simulink model "MCVT_Integration"  (R2020a)
settings: Solver=ode8  StartTime=0.0  StopTime=T_end  FixedStep=dt  RelTol=1e-3  EnableMultiTasking=on
values from neighbouring files (symbols this model uses):
  assumed_power_voltage = 1.25e2    (= 125)    [MCVT_Input_File.m]    (also in MCVT_Run_QuickCheck.m)
  battery_power_toggle = 1    [MCVT_Input_File.m]    (also in MCVT_Run_QuickCheck.m)
  battery_trickle_charge_param = 0.1    [MCVT_Input_File.m]    (also in MCVT_Run_QuickCheck.m)
  battery_trickle_leakage_param = 0.01    [MCVT_Input_File.m]    (also in MCVT_Run_QuickCheck.m)
  damage_case_id = 3    [MCVT_Input_File.m]    (also in MCVT_Run_QuickCheck.m = 1)
  dt = 1/fs    (= 0.001)    [MCVT_Run_QuickCheck.m]    (also in MCVT_Sim_Set.m)
  dt_2 = 1/fs_2    (= 0.001)    [MCVT_Run_QuickCheck.m]    (also in MCVT_Sim_Set.m)
  dt_3 = 1/fs_3    (= 0.001)    [MCVT_Run_QuickCheck.m]    (also in MCVT_Sim_Set.m)
  dt_5 = 1/fs_5    (= 0.005)    [MCVT_Run_QuickCheck.m]    (also in MCVT_Sim_Set.m)
  dt_8 = 1/fs_8    (= 0.05)    [MCVT_Run_QuickCheck.m]    (also in MCVT_Sim_Set.m)
  dt_9 = 1/fs_9    (= 0.001)    [MCVT_Run_QuickCheck.m]    (also in MCVT_Sim_Set.m)
  dust_met_duration = 3    [MCVT_Input_File.m]    (also in MCVT_Run_QuickCheck.m = 2)
  ECLSS_indoor_set_temperature = 298.15    [MCVT_Input_File.m]    (also in MCVT_Run_QuickCheck.m)
  energy_store_max_o = 150    [MCVT_Input_File.m]    (also in MCVT_Run_QuickCheck.m)
  energy_store_o = 120    [MCVT_Input_File.m]    (also in MCVT_Run_QuickCheck.m)
  fs = 1000    [MCVT_Run_QuickCheck.m]    (also in MCVT_Sim_Set.m)
  gain_charge_param = 1    [MCVT_Input_File.m]    (also in MCVT_Run_QuickCheck.m)
  ground_temperature = 373    [MCVT_Habitat_Design_Input_File.m]    (also in MCVT_Run_QuickCheck.m)
  I_max_charge = 800    [MCVT_Input_File.m]    (also in MCVT_Run_QuickCheck.m)
  I_max_discharge = 800    [MCVT_Input_File.m]    (also in MCVT_Run_QuickCheck.m)
  initial_pressure = 1e5    (= 100000)    [MCVT_Input_File.m]    (also in MCVT_Run_QuickCheck.m)
  initial_temperature = 295    [MCVT_Input_File.m]    (also in MCVT_Run_QuickCheck.m)
  interior_leak_rate = 0.00415    [MCVT_Input_File.m]    (also in MCVT_Run_QuickCheck.m)
  launch_dust_accum_start_time = 10    [MCVT_Input_File.m]    (also in MCVT_Run_QuickCheck.m = 2)
  launch_dust_accum_stop_time = 12    [MCVT_Input_File.m]    (also in MCVT_Run_QuickCheck.m = 3)
  meteorite_impact_time = 2    [MCVT_Input_File.m]    (also in MCVT_Run_QuickCheck.m = 1)
  nuclear_power_toggle = 1    [MCVT_Input_File.m]    (also in MCVT_Run_QuickCheck.m)
  print_interval = 30    [MCVT_Input_File.m]
  SOC_lower_thres = 0.5    [MCVT_Input_File.m]    (also in MCVT_Run_QuickCheck.m)
  SOC_upper_thres = 0.9    [MCVT_Input_File.m]    (also in MCVT_Run_QuickCheck.m)
  solar_power_toggle = 1    [MCVT_Input_File.m]    (also in MCVT_Run_QuickCheck.m)
  space_temperature = 4    [MCVT_Habitat_Design_Input_File.m]    (also in MCVT_Run_QuickCheck.m)
  Start_angle = 90    [MCVT_Input_File.m]    (also in MCVT_Run_QuickCheck.m)
undefined symbols (not defined in the file or in its folder): A_flow_restrictor, ACTIVITY_LOOKUP_TABLE, air, Aref, Bmap, Bmap2, Bmap3, comms, DiscIntNeverNeededParam, DmgSPL, dust_parameters, ECLSS_dust_damage_repair_time, ECLSS_heater_capacity, ECLSS_IPS_restrictor, ECLSS_IPS_setpointPressure, ECLSS_paint_damage_repair_time, ECLSS_radiatorLoop_discreteNumber, ECLSS_Vst, emptyActivity, expected_heat_add, FI, habitat, HIEM2SMM, HIEM2STM, HIEMDamageElements, impact_numbering, initial_SPLT_damage_index, input_air, input_Cond, input_CP, input_discretization, input_EV, input_Evap, input_radiatorLoop, K_ECLSS_dust_single, K_ECLSS_paint_single, K_Structure_damage_single, Kref, kW_to_W, meteoroidDustDep, mFRF, ndelays, nominalDustDep, noverlap, nuclear_toggle_gain, output_limits, panel_paint0, Ps0, reference_temperature, RepairTime_ECLSS_factor, Sensor_Location_SMM, SMM_T0, SMM_T1, SMM_T2, SMM_T3, SMM_T4, SMM_T4_0, SMM_T4_1, SMM_T4_2, solar_FDD_K ... (+73)
libraries: neural x350, simulink x39, xpcutilitieslib x7, xpcrtudplib x7, dspstat3 x1
(part 2 of 43)

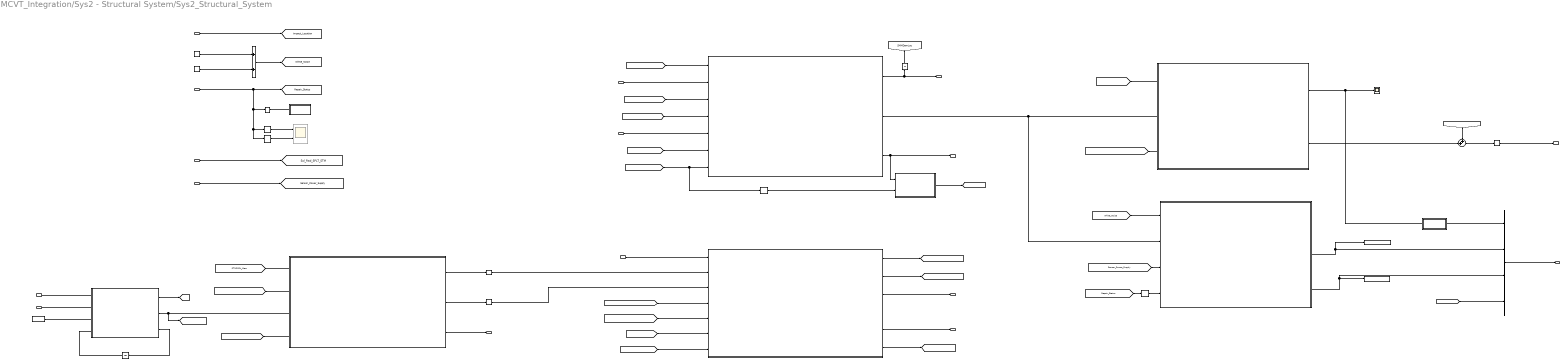
== system MCVT_Integration/Sys2 - Structural System/Sys2_Structural_System ==
  note: Structural Protective Layer (2.4) - Soon it will become its own subsystem block
  note: FDD: Fault Detection and Diagnosis (2.3)
  note: Structural Thermal Model (2.2)
  note: Structural Mechanical Model (2.1)
  note: Input Distribution
  Sys2_In_Solar_Irradiation [W/m^2] : Inport
  Sys2_In_Moonquake_Acceleration [m/s^2] : Inport  Port=2
  Sys2_In_Meteoroid_Location [m] : Inport  Port=3
  Sys2_In_FI_Index [-] : Inport  Port=4
  Sys2_In_dmg_case [-] : Inport  Port=5
  Sys2_In_Interior_Surface_Temperature [K] : Inport  Port=6
  Sys2_In_Internal_Pressure [Pa] : Inport  Port=7
  Sys2_In_Power_Supply [kW] : Inport  Port=8
  Sys2_In_Repair_Status [-] : Inport  Port=9
  3D Structural Protective Layer-Thermal (SPL-T) : Subsystem
  Acc Excitation : simulink/Sources/Band-Limited White Noise  Cov=[1e-9]  Ts=Time_step  seed=[23341]
  Add : Sum
  Bus Creator : BusCreator  Inputs=4
  Derivative1 : Derivative
  FDD adjustment : Subsystem
  Fault Detection and Diagnosis (FDD) 2.3 : Subsystem
  Fault Detection and Diagnosis (FDD) with Binary Classifer : Subsystem
  Impact_Force_&_SPL_Damage : MATLAB Function  TreatAsAtomicUnit=on
  MemBlock : Memory  InitialCondition=initial_SPLT_damage_index
  Memory : Memory  InitialCondition=0.0036
  Repair Status : Constant  Value=0
  SPL2STM_Mem : Memory  InitialCondition=293.15.*ones(10,6)
  STM2SPL_Mem : Memory  InitialCondition=293.15.*ones(10,6)
  Scope4 : Scope
  Selector : Selector  InputPortWidth=4  IndexOptions=Index vector (dialog)  Indices=1  OutputSizes=1
  Selector1 : Selector  InputPortWidth=4  IndexOptions=Index vector (dialog)  Indices=2  OutputSizes=1
  Selector2 : Selector  InputPortWidth=4  IndexOptions=Index vector (dialog)  Indices=3  OutputSizes=1
  Selector3 : Selector  InputPortWidth=4  IndexOptions=Index vector (dialog)  Indices=idx_Activity_Completion_Flag  OutputSizes=1
  Sim FDD Damage Detection : Subsystem
  Structural Mechanical 2.1 : Subsystem
  Structural Thermal Model 2.2 : Subsystem
  Sum : Sum  Inputs=++|
  To Workspace : ToWorkspace  VariableName=DamageProbabilities  SaveFormat=Timeseries  SampleTime=-1
  To Workspace1 : ToWorkspace  VariableName=ImpactProbabilities  SaveFormat=Timeseries  SampleTime=-1
  Unit Delay : UnitDelay  InitialCondition=zeros(324,1)  SampleTime=-1  HasFrameUpgradeWarning=on
  Unit Delay1 : UnitDelay  SampleTime=-1  HasFrameUpgradeWarning=on
  White Noise : simulink/Sources/Band-Limited White Noise  Cov=Noise_power  Ts=Time_step  seed=[23341]
  printRepair : MATLAB Function  TreatAsAtomicUnit=on
  repair : Scope  Commented=on  NumInputPorts=2
  Sys2_out_InteriorWallSurfaceTemp_Sensors [K] : Outport
  Sys2_Out_Damage_Information [-] : Outport  Port=2
  Sys2_out_PowerConsumption [kW] : Outport  Port=3
  Sys2_out_InteriorWallSurfaceTemp [K] : Outport  Port=4  PortDimensions=[60 1]
  Sys2_out_Damage [-] : Outport  Port=5
  Sys2_out_ExteriorWallTemp [K] : Outport  Port=6
  Sys2_out_Total_Damage [-] : Outport  Port=7
  tag scope: ImpForce=global, Impact_Location=global, Repair_Status=global, SMMDamLoc=global, SPL_Dam=global, STM2SMM=global, STM2SPL_Mem=global, Sensor_Power_Supply=global, Sol_Rad_SPLT_STM=global, Tot_Pow_Con=global, White_Noise=global
  wires:
  Acc Excitation/1 -> Add/1
  White Noise/1 -> Add/2
  Structural Mechanical 2.1/2(Sys2.1_Out_Acceleration) -> Fault Detection and Diagnosis (FDD) with Binary Classifer/2(Sys2.3_In_Acceleration Response), Fault Detection and Diagnosis (FDD) 2.3/2(Sys2.3_In_Acceleration Response)
  Sys2_In_Internal_Pressure [Pa]/1 -> Structural Mechanical 2.1/2(Sys2.1_In_Internal_Pressure)
  Sys2_In_Moonquake_Acceleration [m/s^2]/1 -> Structural Mechanical 2.1/5(Sys2.1_In_Moonquake_Acceleration)
  Sum/1 -> Memory/1
  Fault Detection and Diagnosis (FDD) 2.3/1(Sys2.3_Out_Probabilities) -> FDD adjustment/1(x), Scope4/1
  Fault Detection and Diagnosis (FDD) 2.3/2(Sys2.3_Out_Accelerometer Power Consumption (kW)) -> Sum/2
  Structural Mechanical 2.1/1(Sys2.1_Out_Damage_Locations) -> Sys2_out_Damage [-]/1, Unit Delay/1
  Sys2_In_FI_Index [-]/1 -> Impact_Force_&_SPL_Damage/1(FI_index)
  Sys2_In_dmg_case [-]/1 -> Impact_Force_&_SPL_Damage/2(dmg_case)
  Impact_Force_&_SPL_Damage/3(DmgHist) -> Unit Delay1/1
  Unit Delay1/1 -> Impact_Force_&_SPL_Damage/4("DmgHist ")
  Repair Status/1 -> Impact_Force_&_SPL_Damage/3(repair_status)
  Derivative1/1 -> printRepair/1(x)
  Memory/1 -> Sys2_out_PowerConsumption [kW]/1
  Structural Mechanical 2.1/3(Sys2.1_Out_Total_Damage) -> Sim FDD Damage Detection/1(Sys2_Total_Damage), Sys2_out_Total_Damage [-]/1
  FDD adjustment/1(y) -> Bus Creator/1  "Sys2_Out_PanelDamageProbabilities"
  Bus Creator/1 -> Sys2_Out_Damage_Information [-]/1  "Sys2_Out_Damage_Information_to_Sys6"
  Fault Detection and Diagnosis (FDD) with Binary Classifer/3(Sys2.3_Out_Impact_location_Probabilities) -> To Workspace1/1, Bus Creator/3  "Sys2_Out_Impact_Location_Probabiilities"
  Fault Detection and Diagnosis (FDD) with Binary Classifer/2(Sys2.3_Out_Damage_Detection_Probabilities) -> To Workspace/1, Bus Creator/2  "Sys2_Out_Damage_Detection_Probabilities"
  Selector/1 -> Fault Detection and Diagnosis (FDD) with Binary Classifer/4(Sys2.3_In_Repair_Status)
  Sys2_In_Repair_Status [-]/1 -> Selector2/1, Selector1/1, Derivative1/1, [Repair_Status]
  Selector1/1 -> repair/1
  Selector2/1 -> repair/2
  Sys2_In_Meteoroid_Location [m]/1 -> [Impact_Location]
  Structural Thermal Model 2.2/3(Sys2.2_out_InteriorWallSurfTemp_Sensors) -> Sys2_out_InteriorWallSurfaceTemp_Sensors [K]/1
  Structural Thermal Model 2.2/5(Sys2.2_out_InteriorWallSurfaceTemp_(to_Sys8)) -> Sys2_out_InteriorWallSurfaceTemp [K]/1
  3D Structural Protective Layer-Thermal (SPL-T)/1(Sys2.4_SPL2STM_MemoryBlock) -> SPL2STM_Mem/1
  3D Structural Protective Layer-Thermal (SPL-T)/3(Sys2.4_out_ExteriorWallTemp) -> Sys2_out_ExteriorWallTemp [K]/1
  Sys2_In_Interior_Surface_Temperature [K]/1 -> Structural Thermal Model 2.2/1(Sys2.2_In_Interior_Surface_Temperature_(from_Sys8))
  Unit Delay/1 -> [SMMDamLoc]
  [SMMDamLoc] -> Structural Thermal Model 2.2/7(Sys2.2_In_HabitatWallDamage)
  Structural Thermal Model 2.2/6(Sys2.2_out_WallTempDistribution_toSMM (To Sys 2.1)) -> [STM2SMM]
  [STM2SMM] -> Structural Mechanical 2.1/1(Sys2.1_In_Temp_Profile)
  Impact_Force_&_SPL_Damage/2(Dmg) -> 3D Structural Protective Layer-Thermal (SPL-T)/3(Sys2.4_In_Damage_SPL), [SPL_Dam]
  [SPL_Dam] -> Structural Thermal Model 2.2/6(Sys2.2_In_Damage_SPL)
  [Impact_Location] -> 3D Structural Protective Layer-Thermal (SPL-T)/4(Sys2.4_In_Meteorite_Impact_Location)
  Impact_Force_&_SPL_Damage/1(Fimp) -> [ImpForce]
  [ImpForce] -> Structural Mechanical 2.1/3(Sys2.1_In_Impact_Force)
  Sys2_In_Power_Supply [kW]/1 -> [Sensor_Power_Supply]
  [Sensor_Power_Supply] -> Structural Thermal Model 2.2/5(Sys2.2_In_SensorPowerSupply)
  [Sensor_Power_Supply] -> Fault Detection and Diagnosis (FDD) 2.3/3(Sys2.3_In_Accelerometer Power Supply (kW))
  [Sensor_Power_Supply] -> Fault Detection and Diagnosis (FDD) with Binary Classifer/3(Sys2.3_In_Accelerometer Power Supply (kW))
  3D Structural Protective Layer-Thermal (SPL-T)/2(Sys2.4_DamageElementNumber_SPLT_to_STM) -> MemBlock/1
  Sys2_In_Solar_Irradiation [W/m^2]/1 -> [Sol_Rad_SPLT_STM]
  [Sol_Rad_SPLT_STM] -> Structural Thermal Model 2.2/4(Sys2.2_In_Solar_Irradiation_(from_Sys9))
  [Sol_Rad_SPLT_STM] -> 3D Structural Protective Layer-Thermal (SPL-T)/2(Sys2.4_In_Solar_Irradiation_(from_Sys9))
  Structural Thermal Model 2.2/2(Sys2.2_STM2SPL_Mem) -> [STM2SPL_Mem]
  [STM2SPL_Mem] -> 3D Structural Protective Layer-Thermal (SPL-T)/1(Sys2.4_STM2SPL_MemoryBock)
  SPL2STM_Mem/1 -> Structural Thermal Model 2.2/2(Sys2.2_SPL2STM_Mem)
  [Tot_Pow_Con] -> Sum/1
  Structural Thermal Model 2.2/1(Sys2.2_out_TotalPowerConsumption (kW)) -> [Tot_Pow_Con]
  Add/1 -> [White_Noise]
  [White_Noise] -> Structural Mechanical 2.1/6(Sys2.1_In_White Noise)
  [White_Noise] -> Fault Detection and Diagnosis (FDD) 2.3/1(Sys2.3_In_Excitation (White Noise))
  [White_Noise] -> Fault Detection and Diagnosis (FDD) with Binary Classifer/1(Sys2.3_In_Excitation (White Noise))
  [Repair_Status] -> Selector3/1, Structural Mechanical 2.1/7(Sys2.1_In_Repair_Status)
  [Repair_Status] -> Selector/1
  [Impact_Location] -> Structural Mechanical 2.1/4(Sys_2.1_In_Impact_Location)
  MemBlock/1 -> Structural Thermal Model 2.2/3(Sys2.2_Damage_Index_SPLT)
  Selector3/1 -> Sim FDD Damage Detection/2(EnableResetFDD_Structure)
  Sim FDD Damage Detection/1(FDD_Structure_Damage_xe) -> [FDD_Structure_Damage_xe]  "FDD_Structure_Damage_xe"
  [FDD_Structure_Damage_xe] -> Bus Creator/4  "Sys2_Out_FDD_Structure_Damage_xe"

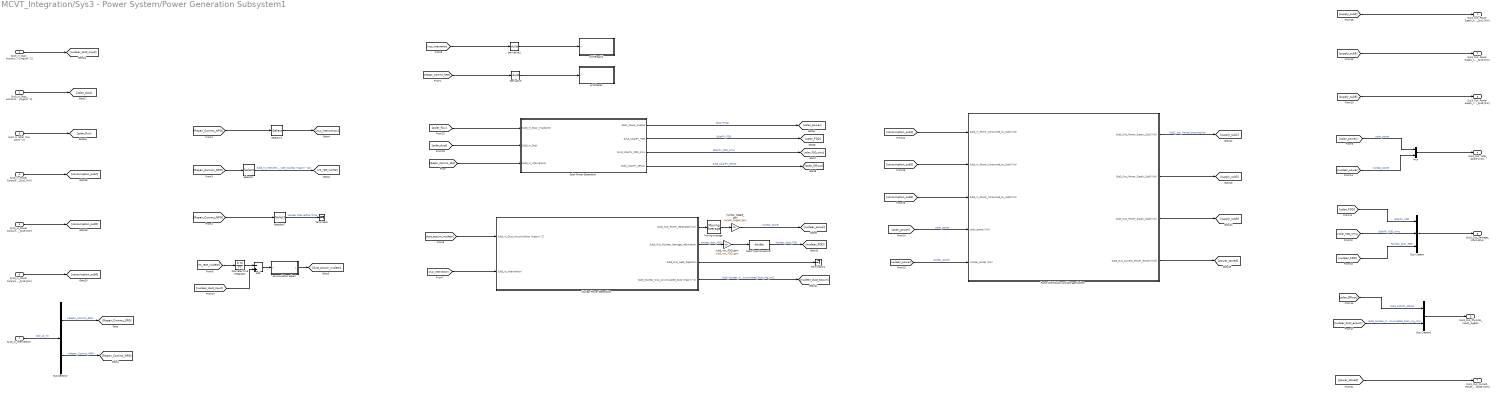
== system MCVT_Integration/Sys3 - Power System/Power Generation Subsystem1 ==
  Sys3_In_Dust_Accumulation_Nuclear [mg/cm^2] : Inport
  Sys3_In_Dust_Accumulation_PV [mg/cm^2] : Inport  Port=2
  Sys3_In_Solar_Flux [W/m^2] : Inport  Port=3
  Sys3_In_Power_Consumed_by_Sys2 [kW] : Inport  Port=4
  Sys3_In_Power_Consumed_by_Sys5 [kW] : Inport  Port=5
  Sys3_In_Power_Consumed_by_Sys8 [kW] : Inport  Port=6
  Sys3_In_Intervention : Inport  Port=7
  Add : Sum  Inputs=-+
  Bus Creator : BusCreator  Inputs=3
  Bus Creator1 : BusCreator  Inputs=2
  Bus Selector : BusSelector  OutputSignals=Repair_Comms_SPG,Repair_Comms_NPG
  Data Type Conversion : DataTypeConversion  OutDataTypeStr=double
  Derivative : Derivative
  Derivative1 : Derivative
  Discrete-Time Integrator : DiscreteIntegrator  SampleTime=-1  ICPrevOutput=DiscIntNeverNeededParam  ICPrevScaledInput=DiscIntNeverNeededParam
  Moving Average : dspstat3/Moving Average  Method=Sliding window  ForgettingFactorPort=off  SpecifyWindowLength=on  WindowLength=1000  ForgettingFactor=0.9  SimulateUsing=Code generation
  Mux : Mux  Inputs=2
  Nuclear Power Generation : Subsystem
  Power Distribution and Storage System : Subsystem
  Selector : Selector  InputPortWidth=4  IndexOptions=Index vector (dialog)  Indices=idx_repair_action_rate  OutputSizes=1
  Selector1 : Selector  InputPortWidth=4  IndexOptions=Index vector (dialog)  Indices=idx_failure_mode  OutputSizes=1
  Selector2 : Selector  InputPortWidth=4  IndexOptions=Index vector (dialog)  Indices=idx_repair_action_time  OutputSizes=1
  Solar Power Generation : Subsystem
  Terminator : Terminator
  Terminator1 : Terminator
  accumulation adjust : MATLAB Function  TreatAsAtomicUnit=on  SystemSampleTime=dt_3
  nuclear_toggle_gain : Gain  Gain=nuclear_toggle_gain
  printRepair : MATLAB Function  TreatAsAtomicUnit=on
  printRepair1 : MATLAB Function  TreatAsAtomicUnit=on
  sub3_nuc_FDD_gain : Gain  Gain=sub3_nuc_FDD_gain
  Sys3_Out_Power_Supply_to_Sys2 [kW] : Outport
  Sys3_Out_Power_Supply_to_Sys5 [kW] : Outport  Port=2
  Sys3_Out_Power_Supply_to_Sys8 [kW] : Outport  Port=3
  Sys3_Out_total_power [kW] : Outport  Port=4
  Sys3_Out_Physical_Inputs_Agents : Outport  Port=5
  Sys3_Out_Damage_Information : Outport  Port=6
  Sys3_Out_Current_Power_Stored [kWh] : Outport  Port=7
  wires:
  Sys3_In_Solar_Flux [W/m^2]/1 -> [solar_flux]
  Sys3_In_Power_Consumed_by_Sys5 [kW]/1 -> [consumption_sub5]
  [supply_sub5] -> Sys3_Out_Power_Supply_to_Sys5 [kW]/1
  Solar Power Generation/1(Solar_Power_Output) -> [solar_power]  "Solar Pmpp"
  Nuclear Power Generation/1(Sub3_Out_Power_Generated (kW)) -> Moving Average/1
  Nuclear Power Generation/3(Sub3_Out_Heat_Rejection) -> Terminator1/1
  Moving Average/1 -> nuclear_toggle_gain/1
  [nuclear_power] -> Mux/2  "nuclear_power"
  Bus Creator/1 -> Sys3_Out_Damage_Information/1
  [solar_FDD] -> Bus Creator/1  "SolarPV_FDD"
  Mux/1 -> Sys3_Out_total_power [kW]/1
  Sys3_In_Dust_Accumulation_PV [mg/cm^2]/1 -> [solar_dust]
  [solar_power] -> Mux/1  "solar_power"
  Bus Selector/1 -> [Repair_Comms_SPG]  "<Repair_Comms_SPG>"
  Derivative/1 -> printRepair/1(x)
  Derivative1/1 -> printRepair1/1(x)
  Sys3_In_Power_Consumed_by_Sys8 [kW]/1 -> [consumption_sub8]
  [supply_sub8] -> Sys3_Out_Power_Supply_to_Sys8 [kW]/1
  Sys3_In_Power_Consumed_by_Sys2 [kW]/1 -> [consumption_sub2]
  [supply_sub2] -> Sys3_Out_Power_Supply_to_Sys2 [kW]/1
  [power_stored] -> Sys3_Out_Current_Power_Stored [kWh]/1
  Nuclear Power Generation/2(Sub3_Out_Nuclear_Damage_Information) -> sub3_nuc_FDD_gain/1  "nuclear_dust_FDD"
  [nuclear_FDD] -> Bus Creator/3  "Nuclear_Dust_FDD"
  sub3_nuc_FDD_gain/1 -> Data Type Conversion/1
  Sys3_In_Dust_Accumulation_Nuclear [mg/cm^2]/1 -> [nuclear_dust_input]
  [nuc_intervenion] -> Nuclear Power Generation/2(Sub3_In_Intervention)
  accumulation adjust/1(y) -> [dust_accum_nuclear]
  [solar_DRout] -> Bus Creator1/1  "Sub3_solarPV_DRout"
  Bus Creator1/1 -> Sys3_Out_Physical_Inputs_Agents/1
  [nuclear_dust_accum] -> Bus Creator1/2  "Sub3_Nuclear_Out_Accumulated_Dust_mg_cm2"
  Selector/1 -> [Int_rate_nuclear]  "Sub3_In_Intervention_rate_Nuclear (mg/cm^2/s)"
  [Repair_Comms_NPG] -> Selector2/1
  Sys3_In_Intervention/1 -> Bus Selector/1  "look_at_int"
  [solar_FDD_simu] -> Bus Creator/2  "SolarPV_FDD_simu"
  Selector2/1 -> Terminator/1  "Nuclear Intervention Time"
  Discrete-Time Integrator/1 -> Add/1
  Add/1 -> accumulation adjust/1(x)
  [Repair_Comms_SPG] -> Solar Power Generation/3(Sub3_In_intervension)
  [Repair_Comms_SPG] -> Derivative/1
  Bus Selector/2 -> [Repair_Comms_NPG]  "<Repair_Comms_NPG>"
  [Repair_Comms_NPG] -> Selector/1
  [Repair_Comms_NPG] -> Selector1/1
  [Int_rate_nuclear] -> Discrete-Time Integrator/1
  [dust_accum_nuclear] -> Nuclear Power Generation/1(Sub3_In_Dust_Accumulation (mg/cm^2))
  Selector1/1 -> [nuc_intervenion]
  [nuc_intervenion] -> Derivative1/1
  [solar_power] -> Power Distribution and Storage System/4(solar_power (kW))  "solar_power"
  nuclear_toggle_gain/1 -> [nuclear_power]  "nuclear_power"
  [nuclear_power] -> Power Distribution and Storage System/5(nuclear_power (kW))  "nuclear_power"
  Data Type Conversion/1 -> [nuclear_FDD]  "Nuclear_Dust_FDD"
  Nuclear Power Generation/4(Sub3_Nuclear_Out_Accumulated_Dust (mg/cm^2)) -> [nuclear_dust_accum]  "Sub3_Nuclear_Out_Accumulated_Dust_mg_cm2"
  Power Distribution and Storage System/1(Sub3_Out_Power_Supply_Sub2 (kW)) -> [supply_sub2]  "Sub2_ out_ PowerConsumpstion"
  Power Distribution and Storage System/2(Sub3_Out_Power_Supply_Sub5 (kW)) -> [supply_sub5]
  Power Distribution and Storage System/3(Sub3_Out_Power_Supply_Sub8 (kW)) -> [supply_sub8]
  Power Distribution and Storage System/4(Sub3_Out_Current_Power_Stored (kWh)) -> [power_stored]
  [solar_flux] -> Solar Power Generation/1(Sub3_In_Solar_Irradiance)
  [solar_dust] -> Solar Power Generation/2(Sub3_In_Dust)
  [consumption_sub2] -> Power Distribution and Storage System/1(Sub3_In_Power_Consumed_by_Sub2 (kW))
  [consumption_sub5] -> Power Distribution and Storage System/2(Sub3_In_Power_Consumed_by_Sub5 (kW))
  [consumption_sub8] -> Power Distribution and Storage System/3(Sub3_In_Power_Consumed_by_Sub8 (kW))
  [nuclear_dust_input] -> Add/2
  Solar Power Generation/2(Sys3_solarPV_FDD) -> [solar_FDD]  "SolarPV_FDD"
  Solar Power Generation/3(Sys3_solarPV_FDD_simu) -> [solar_FDD_simu]  "SolarPV_FDD_simu"
  Solar Power Generation/4(Sub3_solarPV_DRout) -> [solar_DRout]  "Sub3_solarPV_DRout"

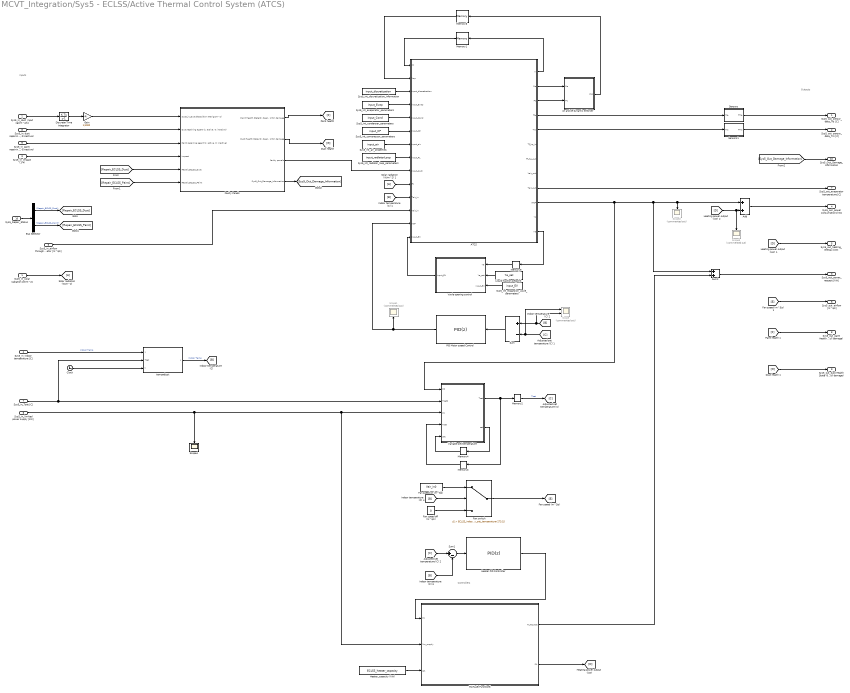
== system MCVT_Integration/Sys5 - ECLSS/Active Thermal Control System (ATCS) ==
  note: Inputs
  note: Outputs
  note: Controllers
  Sys5_in_solar radiation [W/m^2] : Inport
  Sys5_in_dust_Input [g/cm^2/s] : Inport  Port=2
  Sys5_in_impact (1-4) : Inport  Port=3
  Sys5_in_indoor temperature [C] : Inport  Port=4
  Sys5_in_airflow through the evaporator [m^3/s] : Inport  Port=5
  Sys5_in_limited power supply [kW] : Inport  Port=6
  Sys5_in_Tset [C] : Inport  Port=7
  "Sys5_in_dust repairing agent (1:active, 0:inactive)" : Inport  Port=8
  "Sys5_in_paint repairing agent (1:active, 0:inactive)" : Inport  Port=9
  Sys5_Repair_Status : Inport  Port=10
  ATCS : MATLAB Function  TreatAsAtomicUnit=on  SystemSampleTime=dt_5
  Add : Sum
  Bus Selector : BusSelector  OutputSignals=Repair_ECLSS_Dust,Repair_ECLSS_Paint
  Clock : Clock
  Discrete-Time Integrator : DiscreteIntegrator  SampleTime=-1  ICPrevOutput=DiscIntNeverNeededParam  ICPrevScaledInput=DiscIntNeverNeededParam
  Fan speed off (m^3/s) : Constant  Value=0  OutDataTypeStr=Inherit: Inherit via back propagation
  Fan speed on (m^3/s) : Constant  Value=Vair_in0
  Fan switch : Switch  Criteria=u2 > Threshold  Threshold=ECLSS_indoor_set_temperature-273.15
  Faulty Panels : Subsystem
  Gain : Gain  Gain=1/1000
  Heater PID Controller : simulink/Continuous/PID Controller  Controller=PID  Form=Parallel  TimeDomain=Discrete-time  UseExternalTs=off  SampleTime=dt_5  IntegratorMethod=Forward Euler  FilterMethod=Forward Euler  ControllerParametersSource=internal  P=30  I=5  D=0  UseFilter=on  N=100  TunerSelectOption=Transfer Function Based (PID Tuner App)  InitialConditionSource=internal  InitialConditionForIntegrator=0  InitialConditionForFilter=0  DifferentiatorICPrevScaledInput=0  ExternalReset=none  TrackingMode=off  Kt=1  LimitOutput=on  UpperSaturationLimit=inf  LowerSaturationLimit=0  LinearizeAsGain=on  AntiWindupMode=none  Kb=1  POutMin=[]  POutMax=[]  IOutMin=[]  IOutMax=[]  DOutMin=[]  DOutMax=[]  NOutMin=[]  NOutMax=[]  SumOutMin=[]  SumOutMax=[]  SaturationOutMin=[]  SaturationOutMax=[]  PParamMin=[]  PParamMax=[]  IParamMin=[]  IParamMax=[]  DParamMin=[]  DParamMax=[]  NParamMin=[]  NParamMax=[]  KbParamMin=[]  KbParamMax=[]  KtParamMin=[]  KtParamMax=[]  KbOutMin=[]  KbOutMax=[]  KtOutMin=[]  KtOutMax=[]  IntegratorOutMin=[]  IntegratorOutMax=[]  FilterOutMin=[]  FilterOutMax=[]  SumI1OutMin=[]  SumI1OutMax=[]  SumI2OutMin=[]  SumI2OutMax=[]  SumI3OutMin=[]  SumI3OutMax=[]  SumI4OutMin=[]  SumI4OutMax=[]  SumDOutMin=[]  SumDOutMax=[]  DifferentiatorOutMin=[]  DifferentiatorOutMax=[]  FilterDiffOutCoefMin=[]  FilterDiffOutCoefMax=[]  ReciprocalOutMin=[]  ReciprocalOutMax=[]  SumDenOutMin=[]  SumDenOutMax=[]  SumNumOutMin=[]  SumNumOutMax=[]  DivideOutMin=[]  DivideOutMax=[]  UdiffTsProdOutMin=[]  UdiffTsProdOutMax=[]  NTsProdOutMin=[]  NTsProdOutMax=[]  UintegralTsProdOutMin=[]  UintegralTsProdOutMax=[]  UngainTsProdOutMin=[]  UngainTsProdOutMax=[]  IntegratorContinuousStateAttributes=''  IntegratorStateMustResolveToSignalObject=off  IntegratorRTWStateStorageClass=Auto  FilterContinuousStateAttributes=''  FilterStateMustResolveToSignalObject=off  FilterRTWStateStorageClass=Auto  ParallelPVariant=InternalParameters  IdealPVariant=Disabled  IVariant=InternalParameters  DVariant=InternalParameters  IntegratorVariant=Discrete  SatVariant=Enabled  AWVariant=Passthrough  PCopyVariant=Disabled  TRVariant=Disabled  FdbkBlocksVariant=Forward  IdealPFdbkVariant=Disabled  SatFdbkVariant=Disabled  DerivativeFilterVariant=ForwardEulerFilter  NVariant=InternalParameters  NCopyVariant=Disabled  FilterICVariant=InternalICsFilter  IntegratorICVariant=InternalICs  ExternalResetVariant=Disabled  TRSumVariant=Passthrough  SumFdbkVariant=Disabled  SumVariant=Sum_PID  TsampFilterVariant=InternalTs  TsampNgainVariant=Passthrough  TsampIntegralVariant=Passthrough
  Heater_capacity (kW) : Constant  Value=ECLSS_heater_capacity
  Liquid fraction setpoint : Constant  Value=Ye_set
  MATLAB Function6 : MATLAB Function  TreatAsAtomicUnit=on
  Memory1 : Memory  InitialCondition=X0_ATC  InheritSampleTime=on
  Memory2 : Memory  InitialCondition=Tset0  InheritSampleTime=on
  Memory3 : Memory  InitialCondition=Tset0  InheritSampleTime=on
  Memory4 : Memory  InheritSampleTime=on
  Memory6 : Memory  InitialCondition=1  InheritSampleTime=on
  Memory9 : Memory  InitialCondition=Ye0  InheritSampleTime=on